simulink model "CdsnProbltyMgr"  (R2022b)
settings: Solver=FixedStepDiscrete  StartTime=0.0  StopTime=10.0  FixedStep=0.05  RelTol=1e-3
(part 2 of 2)

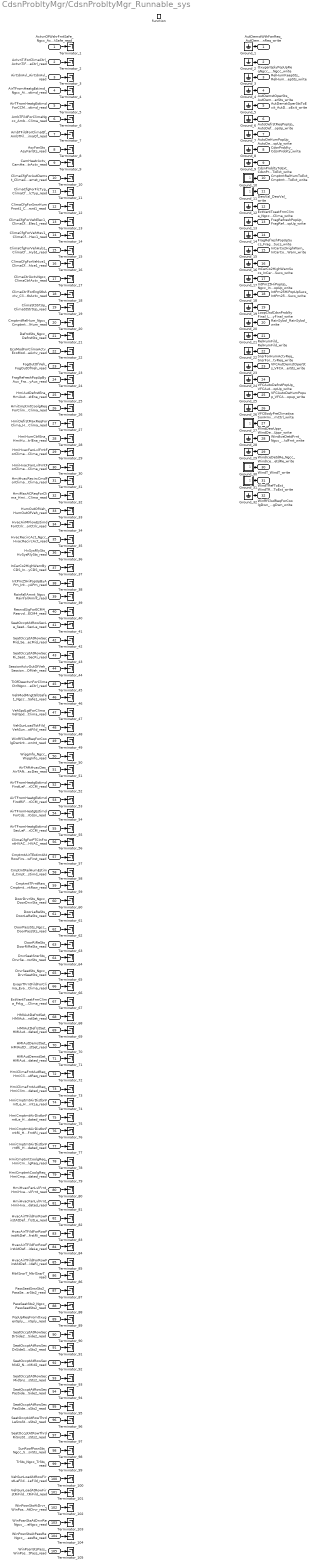
== system CdsnProbltyMgr/CdsnProbltyMgr_Runnable_sys ==
  ActvnOfWshrFrntSafe_Ngcc_ActvnOfWshrFrntSafe_read : Inport
  ActvnTiForClimaCtrl_ActvnTiForClimaCtrl_read : Inport  Port=2
  AirCdnAvl_AirCdnAvl_read : Inport  Port=3
  AirTFromHeatgEstimd_Ngcc_AirTFromHeatgEstimd_read : Inport  Port=4
  AirTFromHeatgEstimdForCCM_AirTFromHeatgEstimd_read : Inport  Port=5
  AmbTFildForClimaNgcc_AmbTFildForClima_read : Inport  Port=6
  AmbTFildForClimaQf_AmbTFildForClimaQf_read : Inport  Port=7
  AsyFanSts_AsyFanSts_read : Inport  Port=8
  CamHeatrActv_CamHeatrActv_read : Inport  Port=9
  ClimaCfgForAutDemst_ClimaCfgForAutDemst_read : Inport  Port=10
  ClimaCfgForFlcTyp_ClimaCfgForFlcTyp_read : Inport  Port=11
  ClimaCfgForSnsrHumPrsnt1_ClimaCfgForSnsrHumPrsnt1_read : Inport  Port=12
  ClimaCfgForVehElec1_ClimaCfgForVehElec1_read : Inport  Port=13
  ClimaCfgForVehHev1_ClimaCfgForVehHev1_read : Inport  Port=14
  ClimaCfgForVehHyb1_ClimaCfgForVehHyb1_read : Inport  Port=15
  ClimaCfgForVehIce1_ClimaCfgForVehIce1_read : Inport  Port=16
  ClimaCtrlActvNgcc_ClimaCtrlActv_read : Inport  Port=17
  ClimaCtrlForEngStbActv_ClimaCtrlForEngStbActv_read : Inport  Port=18
  ClimaStStrtUp_ClimaStStrtUp_read : Inport  Port=19
  CmptmtRelHum_Ngcc_CmptmtRelHum_read : Inport  Port=20
  DefrstSts_Ngcc_DefrstSts_read : Inport  Port=21
  EcoModForClimaActv_EcoModForClimaActv_read : Inport  Port=22
  FogOutOfVeh_FogOutOfVeh_read : Inport  Port=23
  FragRefreshPopUpByAux_FragRefreshPopUpByAux_read : Inport  Port=24
  HmiAutDefrstEna_HmiAutDefrstEna_read : Inport  Port=25
  HmiCmptmtCoolgReqForClima_HmiCmptmtCoolgReqForClima_read : Inport  Port=26
  HmiDefrstMaxReqForClima_HmiDefrstMaxReqForClima_read : Inport  Port=27
  HmiHumCtrlEna_HmiHumCtrlEna_read : Inport  Port=28
  HmiHvacFanLvlFrntForClima_HmiHvacFanLvlFrntForClima_read : Inport  Port=29
  HmiHvacFanLvlFrntForClima_HmiHvacFanLvlFrntRiForClima_read : Inport  Port=30
  HmiHvacRecircCmdForClima_HmiHvacRecircCmdForClima_read : Inport  Port=31
  HmiMaxACReqForClima_HmiMaxACReqForClima_read : Inport  Port=32
  HumOutOfVeh_HumOutOfVeh_read : Inport  Port=33
  HvacAirMFlowEstimdForCtrlr_HvacAirMFlowEstimdForCtrlr_read : Inport  Port=34
  HvacRecircAct_Ngcc_HvacRecircAct_read : Inport  Port=35
  HvSysRlySts_HvSysRlySts_read : Inport  Port=36
  InCarCo2HighWarnByCDS_InCarCo2HighWarnByCDS_read : Inport  Port=37
  IntPm25HiPopUpByAPm_IntPm25HiPopUpByAPm_read : Inport  Port=38
  RainfallAmnt_Ngcc_RainfallAmnt_read : Inport  Port=39
  ResrvdSigForECM4_ResrvdSigForECM4_read : Inport  Port=40
  SeatOccptAtRowSecLe_SeatOccptAtRowSecLe_read : Inport  Port=41
  SeatOccptAtRowSecMid_SeatOccptAtRowSecMid_read : Inport  Port=42
  SeatOccptAtRowSecRi_SeatOccptAtRowSecRi_read : Inport  Port=43
  SessionActvOutOfVeh_SessionActvOutOfVeh_read : Inport  Port=44
  TiOfDeactvnForClimaCtrlNgcc_TiOfDeactvnForClimaCtrl_read : Inport  Port=45
  VehModMngtGlbSafe1_Ngcc_VehModMngtGlbSafe1_read : Inport  Port=46
  VehSpdLgtForClima_VehSpdLgtForClima_read : Inport  Port=47
  VehSunLoadTotFild_VehSunLoadTotFild_read : Inport  Port=48
  WinRfClsdReqForCoolgDwnInt_WinRfClsdReqForCoolgDwnInt_read : Inport  Port=49
  WipgInfo_Ngcc_WipgInfo_read : Inport  Port=50
  AirTAftHvacDes_AirTAftHvacDes_read : Inport  Port=51
  AirTFromHeatgEstimdFirstLeForCCM_AirTFromHeatgEstimdFirstLeForCCM_read : Inport  Port=52
  AirTFromHeatgEstimdFirstRiForCCM_AirTFromHeatgEstimdFirstRiForCCM_read : Inport  Port=53
  AirTFromHeatgEstimdForCdsn_AirTFromHeatgEstimdForCdsn_read : Inport  Port=54
  AirTFromHeatgEstimdSecLeForCCM_AirTFromHeatgEstimdSecLeForCCM_read : Inport  Port=55
  ClimaCfgForPTCInFrontHVAC_ClimaCfgForPTCInFrontHVAC_read : Inport  Port=56
  CmptmtAirTEstimdAtRowFirst_CmptmtAirTEstimdAtRowFirst_read : Inport  Port=57
  CmptmtRelHumEstimd_CmptmtRelHumEstimd_read : Inport  Port=58
  CmptmtTFrntRaw_CmptmtTFrntRaw_read : Inport  Port=59
  DoorDrvrSts_Ngcc_DoorDrvrSts_read : Inport  Port=60
  DoorLeReSts_DoorLeReSts_read : Inport  Port=61
  DoorPassSts_Ngcc_DoorPassSts_read : Inport  Port=62
  DoorRiReSts_DoorRiReSts_read : Inport  Port=63
  DrvrSeatSnsrSts_DrvrSeatSnsrSts_read : Inport  Port=64
  DrvrSeatSts_Ngcc_DrvrSeatSts_read : Inport  Port=65
  EvaprTFrntFildForClima_EvaprTFrntFildForClima_read : Inport  Port=66
  ExtVentToastFrmClima_Prkg_ExtVentToastFrmClima_read : Inport  Port=67
  HMIAutDefrstSet_HMIAutDefrstSet_read : Inport  Port=68
  HMIAutDefrstSet_HMIAutDefrstSet_IsUpdated_read : Inport  Port=69
  HMIAutDemstSet_HMIAutDemstSet_read : Inport  Port=70
  HMIAutDemstSet_HMIAutDemstSet_IsUpdated_read : Inport  Port=71
  HmiClimaFrntAutReq_HmiClimaFrntAutReq_read : Inport  Port=72
  HmiClimaFrntAutReq_HmiClimaFrntAutReq_IsUpdated_read : Inport  Port=73
  HmiCmptmtAirDistbnFrntLe_HmiCmptmtAirDistbnFrntLe_read : Inport  Port=74
  HmiCmptmtAirDistbnFrntLe_HmiCmptmtAirDistbnFrntLe_IsUpdated_read : Inport  Port=75
  HmiCmptmtAirDistbnFrntRi_HmiCmptmtAirDistbnFrntRi_read : Inport  Port=76
  HmiCmptmtAirDistbnFrntRi_HmiCmptmtAirDistbnFrntRi_IsUpdated_read : Inport  Port=77
  HmiCmptmtCoolgReq_HmiCmptmtCoolgReq_read : Inport  Port=78
  HmiCmptmtCoolgReq_HmiCmptmtCoolgReq_IsUpdated_read : Inport  Port=79
  HmiHvacFanLvlFrnt_HmiHvacFanLvlFrnt_read : Inport  Port=80
  HmiHvacFanLvlFrnt_HmiHvacFanLvlFrnt_IsUpdated_read : Inport  Port=81
  HvacAirTFildForRowFirstAtDefrstLe_HvacAirTFildForRowFirstAtDefrstLe_read : Inport  Port=82
  HvacAirTFildForRowFirstAtDefrstRi_HvacAirTFildForRowFirstAtDefrstRi_read : Inport  Port=83
  HvacAirTFildForRowFirstAtDefrstSideLe_HvacAirTFildForRowFirstAtDefrstSideLe_read : Inport  Port=84
  HvacAirTFildForRowFirstAtDefrstSideRi_HvacAirTFildForRowFirstAtDefrstSideRi_read : Inport  Port=85
  MtrlSnsrT_MtrlSnsrT_read : Inport  Port=86
  PassSeatSnsrSts2_PassSeatSnsrSts2_read : Inport  Port=87
  PassSeatSts2_Ngcc_PassSeatSts2_read : Inport  Port=88
  PopUpReqFromOxygenSply_PopUpReqFromOxygenSply_read : Inport  Port=89
  SeatOccptAtRowSecDrSide2_Ngcc_SeatOccptAtRowSecDrSide2_read : Inport  Port=90
  SeatOccptAtRowSecDrSideSnsSts2_SeatOccptAtRowSecDrSideSnsSts2_read : Inport  Port=91
  SeatOccptAtRowSecMid2_Ngcc_SeatOccptAtRowSecMid2_read : Inport  Port=92
  SeatOccptAtRowSecMidSnsSts2_SeatOccptAtRowSecMidSnsSts2_read : Inport  Port=93
  SeatOccptAtRowSecPasSide2_Ngcc_SeatOccptAtRowSecPasSide2_read : Inport  Port=94
  SeatOccptAtRowSecPasSideSnsSts2_SeatOccptAtRowSecPasSideSnsSts2_read : Inport  Port=95
  SeatOccptAtRowThrdLeSnsSts2_SeatOccptAtRowThrdLeSnsSts2_read : Inport  Port=96
  SeatOccptAtRowThrdRiSnsSts2_SeatOccptAtRowThrdRiSnsSts2_read : Inport  Port=97
  SunRoofPosnSts_Ngcc_SunRoofPosnSts_read : Inport  Port=98
  TrSts_Ngcc_TrSts_read : Inport  Port=99
  VehSunLoadAtRowFirstLeFild_VehSunLoadAtRowFirstLeFild_read : Inport  Port=100
  VehSunLoadAtRowFirstRiFild_VehSunLoadAtRowFirstRiFild_read : Inport  Port=101
  WinPosnStsAtDrvr_WinPosnStsAtDrvr_read : Inport  Port=102
  WinPosnStsAtDrvrReNgcc_WinPosnStsAtDrvrReNgcc_read : Inport  Port=103
  WinPosnStsAtPassReNgcc_WinPosnStsAtPassRe_read : Inport  Port=104
  WinPosnStsPass_WinPosnStsAtPass_read : Inport  Port=105
  function : TriggerPort  TriggerType=function-call  VariantControl=(inherit)  SampleTimeType=periodic  SampleTime=-1
  Ground_{1..9,12..26,28..29,32} (27 alike) : Ground
  Ground_10 : Subsystem
  Ground_11 : Subsystem
  Ground_27 : Subsystem
  Ground_30 : Subsystem
  Ground_31 : Subsystem
  Terminator_{1..105} (105 alike) : Terminator
  AutDemstWthFanReq_AutDemstWthFanReq_write : Outport
      settings: EnsureOutportIsVirtual=on
  OxygenSplyPopUpReqNgcc_OxygenSplyPopUpReqNgcc_write : Outport  Port=2
      settings: EnsureOutportIsVirtual=on
  RelHumKeepSts_RelHumKeepSts_write : Outport  Port=3
      settings: EnsureOutportIsVirtual=on
  AutDemstOperSts_AutDemstOperSts_write : Outport  Port=4
      settings: EnsureOutportIsVirtual=on
  AutDemstOperStsToExit_AutDemstOperStsToExit_write : Outport  Port=5
      settings: EnsureOutportIsVirtual=on
  AutoDefrstReqPopUp_AutoDefrstReqPopUp_write : Outport  Port=6
      settings: EnsureOutportIsVirtual=on
  AutoDeHumPopUp_AutoDeHumPopUp_write : Outport  Port=7
      settings: EnsureOutportIsVirtual=on
  CdsnProblty_CdsnProblty_write : Outport  Port=8
      settings: EnsureOutportIsVirtual=on
  CdsnProbltyToExt_CdsnProbltyToExt_write : Outport  Port=9
      settings: EnsureOutportIsVirtual=on
  CmptmtRelHumToExt_CmptmtRelHumToExt_write : Outport  Port=10
      settings: EnsureOutportIsVirtual=on
  DewVal_DewVal_write : Outport  Port=11
      settings: EnsureOutportIsVirtual=on
  ExtVentToastFrmClima_Ngcc_ExtVentToastFrmClima_write : Outport  Port=12
      settings: EnsureOutportIsVirtual=on
  FragRefreshPopUp_FragRefreshPopUp_write : Outport  Port=13
      settings: EnsureOutportIsVirtual=on
  FragRefreshPopUpSucs_FragRefreshPopUpSucs_write : Outport  Port=14
      settings: EnsureOutportIsVirtual=on
  InCarCo2HighWarn_InCarCo2HighWarn_write : Outport  Port=15
      settings: EnsureOutportIsVirtual=on
  InCarCo2HighWarnSucs_InCarCo2HighWarnSucs_write : Outport  Port=16
      settings: EnsureOutportIsVirtual=on
  IntPm25HiPopUp_Ngcc_IntPm25HiPopUp_write : Outport  Port=17
      settings: EnsureOutportIsVirtual=on
  IntPm25HiPopUpSucs_IntPm25HiPopUpSucs_write : Outport  Port=18
      settings: EnsureOutportIsVirtual=on
  LoopClsdCdsnProbltyFinal_LoopClsdCdsnProbltyFinal_write : Outport  Port=19
      settings: EnsureOutportIsVirtual=on
  RainSybol_RainSybol_write : Outport  Port=20
      settings: EnsureOutportIsVirtual=on
  RelHumFild_RelHumFild_write : Outport  Port=21
      settings: EnsureOutportIsVirtual=on
  SnsrForHumActvReq_SnsrForHumActvReq_write : Outport  Port=22
      settings: EnsureOutportIsVirtual=on
  VFCAutDemstOperSts_VFCAutDemstOperSts_write : Outport  Port=23
      settings: EnsureOutportIsVirtual=on
  VFCAutoDefrstPopUp_VFCAutoDefrstPopUp_write : Outport  Port=24
      settings: EnsureOutportIsVirtual=on
  VFCAutoDeHumPopup_VFCAutoDeHumPopup_write : Outport  Port=25
      settings: EnsureOutportIsVirtual=on
  VFCBodyPreClimatisationHmiHumCtrl_VFCBodyPreClimatisationHmiHumCtrl_write : Outport  Port=26
      settings: EnsureOutportIsVirtual=on
  WindDewUppr_WindDewTUppr_write : Outport  Port=27
      settings: EnsureOutportIsVirtual=on
  WindIceDetdFrnt_Ngcc_WindIceDetdFrnt_write : Outport  Port=28
      settings: EnsureOutportIsVirtual=on
  WindIceDetdRe_Ngcc_WindIceDetdRe_write : Outport  Port=29
      settings: EnsureOutportIsVirtual=on
  WindT_WindT_write : Outport  Port=30
      settings: EnsureOutportIsVirtual=on
  WindTRefToExt_WindTRefToExt_write : Outport  Port=31
      settings: EnsureOutportIsVirtual=on
  WinRfClsdReqForCoolgDwn_WinRfClsdReqForCoolgDwn_write : Outport  Port=32
      settings: EnsureOutportIsVirtual=on
  wires:
  ActvnOfWshrFrntSafe_Ngcc_ActvnOfWshrFrntSafe_read/1 -> Terminator_1/1
  ActvnTiForClimaCtrl_ActvnTiForClimaCtrl_read/1 -> Terminator_2/1
  AirCdnAvl_AirCdnAvl_read/1 -> Terminator_3/1
  AirTFromHeatgEstimd_Ngcc_AirTFromHeatgEstimd_read/1 -> Terminator_4/1
  AirTFromHeatgEstimdForCCM_AirTFromHeatgEstimd_read/1 -> Terminator_5/1
  AmbTFildForClimaNgcc_AmbTFildForClima_read/1 -> Terminator_6/1
  AmbTFildForClimaQf_AmbTFildForClimaQf_read/1 -> Terminator_7/1
  AsyFanSts_AsyFanSts_read/1 -> Terminator_8/1
  CamHeatrActv_CamHeatrActv_read/1 -> Terminator_9/1
  ClimaCfgForAutDemst_ClimaCfgForAutDemst_read/1 -> Terminator_10/1
  ClimaCfgForFlcTyp_ClimaCfgForFlcTyp_read/1 -> Terminator_11/1
  ClimaCfgForSnsrHumPrsnt1_ClimaCfgForSnsrHumPrsnt1_read/1 -> Terminator_12/1
  ClimaCfgForVehElec1_ClimaCfgForVehElec1_read/1 -> Terminator_13/1
  ClimaCfgForVehHev1_ClimaCfgForVehHev1_read/1 -> Terminator_14/1
  ClimaCfgForVehHyb1_ClimaCfgForVehHyb1_read/1 -> Terminator_15/1
  ClimaCfgForVehIce1_ClimaCfgForVehIce1_read/1 -> Terminator_16/1
  ClimaCtrlActvNgcc_ClimaCtrlActv_read/1 -> Terminator_17/1
  ClimaCtrlForEngStbActv_ClimaCtrlForEngStbActv_read/1 -> Terminator_18/1
  ClimaStStrtUp_ClimaStStrtUp_read/1 -> Terminator_19/1
  CmptmtRelHum_Ngcc_CmptmtRelHum_read/1 -> Terminator_20/1
  DefrstSts_Ngcc_DefrstSts_read/1 -> Terminator_21/1
  EcoModForClimaActv_EcoModForClimaActv_read/1 -> Terminator_22/1
  FogOutOfVeh_FogOutOfVeh_read/1 -> Terminator_23/1
  FragRefreshPopUpByAux_FragRefreshPopUpByAux_read/1 -> Terminator_24/1
  HmiAutDefrstEna_HmiAutDefrstEna_read/1 -> Terminator_25/1
  HmiCmptmtCoolgReqForClima_HmiCmptmtCoolgReqForClima_read/1 -> Terminator_26/1
  HmiDefrstMaxReqForClima_HmiDefrstMaxReqForClima_read/1 -> Terminator_27/1
  HmiHumCtrlEna_HmiHumCtrlEna_read/1 -> Terminator_28/1
  HmiHvacFanLvlFrntForClima_HmiHvacFanLvlFrntForClima_read/1 -> Terminator_29/1
  HmiHvacFanLvlFrntForClima_HmiHvacFanLvlFrntRiForClima_read/1 -> Terminator_30/1
  HmiHvacRecircCmdForClima_HmiHvacRecircCmdForClima_read/1 -> Terminator_31/1
  HmiMaxACReqForClima_HmiMaxACReqForClima_read/1 -> Terminator_32/1
  HumOutOfVeh_HumOutOfVeh_read/1 -> Terminator_33/1
  HvacAirMFlowEstimdForCtrlr_HvacAirMFlowEstimdForCtrlr_read/1 -> Terminator_34/1
  HvacRecircAct_Ngcc_HvacRecircAct_read/1 -> Terminator_35/1
  HvSysRlySts_HvSysRlySts_read/1 -> Terminator_36/1
  InCarCo2HighWarnByCDS_InCarCo2HighWarnByCDS_read/1 -> Terminator_37/1
  IntPm25HiPopUpByAPm_IntPm25HiPopUpByAPm_read/1 -> Terminator_38/1
  RainfallAmnt_Ngcc_RainfallAmnt_read/1 -> Terminator_39/1
  ResrvdSigForECM4_ResrvdSigForECM4_read/1 -> Terminator_40/1
  SeatOccptAtRowSecLe_SeatOccptAtRowSecLe_read/1 -> Terminator_41/1
  SeatOccptAtRowSecMid_SeatOccptAtRowSecMid_read/1 -> Terminator_42/1
  SeatOccptAtRowSecRi_SeatOccptAtRowSecRi_read/1 -> Terminator_43/1
  SessionActvOutOfVeh_SessionActvOutOfVeh_read/1 -> Terminator_44/1
  TiOfDeactvnForClimaCtrlNgcc_TiOfDeactvnForClimaCtrl_read/1 -> Terminator_45/1
  VehModMngtGlbSafe1_Ngcc_VehModMngtGlbSafe1_read/1 -> Terminator_46/1
  VehSpdLgtForClima_VehSpdLgtForClima_read/1 -> Terminator_47/1
  VehSunLoadTotFild_VehSunLoadTotFild_read/1 -> Terminator_48/1
  WinRfClsdReqForCoolgDwnInt_WinRfClsdReqForCoolgDwnInt_read/1 -> Terminator_49/1
  WipgInfo_Ngcc_WipgInfo_read/1 -> Terminator_50/1
  AirTAftHvacDes_AirTAftHvacDes_read/1 -> Terminator_51/1
  AirTFromHeatgEstimdFirstLeForCCM_AirTFromHeatgEstimdFirstLeForCCM_read/1 -> Terminator_52/1
  AirTFromHeatgEstimdFirstRiForCCM_AirTFromHeatgEstimdFirstRiForCCM_read/1 -> Terminator_53/1
  AirTFromHeatgEstimdForCdsn_AirTFromHeatgEstimdForCdsn_read/1 -> Terminator_54/1
  AirTFromHeatgEstimdSecLeForCCM_AirTFromHeatgEstimdSecLeForCCM_read/1 -> Terminator_55/1
  ClimaCfgForPTCInFrontHVAC_ClimaCfgForPTCInFrontHVAC_read/1 -> Terminator_56/1
  CmptmtAirTEstimdAtRowFirst_CmptmtAirTEstimdAtRowFirst_read/1 -> Terminator_57/1
  CmptmtRelHumEstimd_CmptmtRelHumEstimd_read/1 -> Terminator_58/1
  CmptmtTFrntRaw_CmptmtTFrntRaw_read/1 -> Terminator_59/1
  DoorDrvrSts_Ngcc_DoorDrvrSts_read/1 -> Terminator_60/1
  DoorLeReSts_DoorLeReSts_read/1 -> Terminator_61/1
  DoorPassSts_Ngcc_DoorPassSts_read/1 -> Terminator_62/1
  DoorRiReSts_DoorRiReSts_read/1 -> Terminator_63/1
  DrvrSeatSnsrSts_DrvrSeatSnsrSts_read/1 -> Terminator_64/1
  DrvrSeatSts_Ngcc_DrvrSeatSts_read/1 -> Terminator_65/1
  EvaprTFrntFildForClima_EvaprTFrntFildForClima_read/1 -> Terminator_66/1
  ExtVentToastFrmClima_Prkg_ExtVentToastFrmClima_read/1 -> Terminator_67/1
  HMIAutDefrstSet_HMIAutDefrstSet_read/1 -> Terminator_68/1
  HMIAutDefrstSet_HMIAutDefrstSet_IsUpdated_read/1 -> Terminator_69/1
  HMIAutDemstSet_HMIAutDemstSet_read/1 -> Terminator_70/1
  HMIAutDemstSet_HMIAutDemstSet_IsUpdated_read/1 -> Terminator_71/1
  HmiClimaFrntAutReq_HmiClimaFrntAutReq_read/1 -> Terminator_72/1
  HmiClimaFrntAutReq_HmiClimaFrntAutReq_IsUpdated_read/1 -> Terminator_73/1
  HmiCmptmtAirDistbnFrntLe_HmiCmptmtAirDistbnFrntLe_read/1 -> Terminator_74/1
  HmiCmptmtAirDistbnFrntLe_HmiCmptmtAirDistbnFrntLe_IsUpdated_read/1 -> Terminator_75/1
  HmiCmptmtAirDistbnFrntRi_HmiCmptmtAirDistbnFrntRi_read/1 -> Terminator_76/1
  HmiCmptmtAirDistbnFrntRi_HmiCmptmtAirDistbnFrntRi_IsUpdated_read/1 -> Terminator_77/1
  HmiCmptmtCoolgReq_HmiCmptmtCoolgReq_read/1 -> Terminator_78/1
  HmiCmptmtCoolgReq_HmiCmptmtCoolgReq_IsUpdated_read/1 -> Terminator_79/1
  HmiHvacFanLvlFrnt_HmiHvacFanLvlFrnt_read/1 -> Terminator_80/1
  HmiHvacFanLvlFrnt_HmiHvacFanLvlFrnt_IsUpdated_read/1 -> Terminator_81/1
  HvacAirTFildForRowFirstAtDefrstLe_HvacAirTFildForRowFirstAtDefrstLe_read/1 -> Terminator_82/1
  HvacAirTFildForRowFirstAtDefrstRi_HvacAirTFildForRowFirstAtDefrstRi_read/1 -> Terminator_83/1
  HvacAirTFildForRowFirstAtDefrstSideLe_HvacAirTFildForRowFirstAtDefrstSideLe_read/1 -> Terminator_84/1
  HvacAirTFildForRowFirstAtDefrstSideRi_HvacAirTFildForRowFirstAtDefrstSideRi_read/1 -> Terminator_85/1
  MtrlSnsrT_MtrlSnsrT_read/1 -> Terminator_86/1
  PassSeatSnsrSts2_PassSeatSnsrSts2_read/1 -> Terminator_87/1
  PassSeatSts2_Ngcc_PassSeatSts2_read/1 -> Terminator_88/1
  PopUpReqFromOxygenSply_PopUpReqFromOxygenSply_read/1 -> Terminator_89/1
  SeatOccptAtRowSecDrSide2_Ngcc_SeatOccptAtRowSecDrSide2_read/1 -> Terminator_90/1
  SeatOccptAtRowSecDrSideSnsSts2_SeatOccptAtRowSecDrSideSnsSts2_read/1 -> Terminator_91/1
  SeatOccptAtRowSecMid2_Ngcc_SeatOccptAtRowSecMid2_read/1 -> Terminator_92/1
  SeatOccptAtRowSecMidSnsSts2_SeatOccptAtRowSecMidSnsSts2_read/1 -> Terminator_93/1
  SeatOccptAtRowSecPasSide2_Ngcc_SeatOccptAtRowSecPasSide2_read/1 -> Terminator_94/1
  SeatOccptAtRowSecPasSideSnsSts2_SeatOccptAtRowSecPasSideSnsSts2_read/1 -> Terminator_95/1
  SeatOccptAtRowThrdLeSnsSts2_SeatOccptAtRowThrdLeSnsSts2_read/1 -> Terminator_96/1
  SeatOccptAtRowThrdRiSnsSts2_SeatOccptAtRowThrdRiSnsSts2_read/1 -> Terminator_97/1
  SunRoofPosnSts_Ngcc_SunRoofPosnSts_read/1 -> Terminator_98/1
  TrSts_Ngcc_TrSts_read/1 -> Terminator_99/1
  VehSunLoadAtRowFirstLeFild_VehSunLoadAtRowFirstLeFild_read/1 -> Terminator_100/1
  VehSunLoadAtRowFirstRiFild_VehSunLoadAtRowFirstRiFild_read/1 -> Terminator_101/1
  WinPosnStsAtDrvr_WinPosnStsAtDrvr_read/1 -> Terminator_102/1
  WinPosnStsAtDrvrReNgcc_WinPosnStsAtDrvrReNgcc_read/1 -> Terminator_103/1
  WinPosnStsAtPassReNgcc_WinPosnStsAtPassRe_read/1 -> Terminator_104/1
  WinPosnStsPass_WinPosnStsAtPass_read/1 -> Terminator_105/1
  Ground_1/1 -> AutDemstWthFanReq_AutDemstWthFanReq_write/1
  Ground_2/1 -> OxygenSplyPopUpReqNgcc_OxygenSplyPopUpReqNgcc_write/1
  Ground_3/1 -> RelHumKeepSts_RelHumKeepSts_write/1
  Ground_4/1 -> AutDemstOperSts_AutDemstOperSts_write/1
  Ground_5/1 -> AutDemstOperStsToExit_AutDemstOperStsToExit_write/1
  Ground_6/1 -> AutoDefrstReqPopUp_AutoDefrstReqPopUp_write/1
  Ground_7/1 -> AutoDeHumPopUp_AutoDeHumPopUp_write/1
  Ground_8/1 -> CdsnProblty_CdsnProblty_write/1
  Ground_9/1 -> CdsnProbltyToExt_CdsnProbltyToExt_write/1
  Ground_10/1(Out1) -> CmptmtRelHumToExt_CmptmtRelHumToExt_write/1
  Ground_11/1(Out1) -> DewVal_DewVal_write/1
  Ground_12/1 -> ExtVentToastFrmClima_Ngcc_ExtVentToastFrmClima_write/1
  Ground_13/1 -> FragRefreshPopUp_FragRefreshPopUp_write/1
  Ground_14/1 -> FragRefreshPopUpSucs_FragRefreshPopUpSucs_write/1
  Ground_15/1 -> InCarCo2HighWarn_InCarCo2HighWarn_write/1
  Ground_16/1 -> InCarCo2HighWarnSucs_InCarCo2HighWarnSucs_write/1
  Ground_17/1 -> IntPm25HiPopUp_Ngcc_IntPm25HiPopUp_write/1
  Ground_18/1 -> IntPm25HiPopUpSucs_IntPm25HiPopUpSucs_write/1
  Ground_19/1 -> LoopClsdCdsnProbltyFinal_LoopClsdCdsnProbltyFinal_write/1
  Ground_20/1 -> RainSybol_RainSybol_write/1
  Ground_21/1 -> RelHumFild_RelHumFild_write/1
  Ground_22/1 -> SnsrForHumActvReq_SnsrForHumActvReq_write/1
  Ground_23/1 -> VFCAutDemstOperSts_VFCAutDemstOperSts_write/1
  Ground_24/1 -> VFCAutoDefrstPopUp_VFCAutoDefrstPopUp_write/1
  Ground_25/1 -> VFCAutoDeHumPopup_VFCAutoDeHumPopup_write/1
  Ground_26/1 -> VFCBodyPreClimatisationHmiHumCtrl_VFCBodyPreClimatisationHmiHumCtrl_write/1
  Ground_27/1(Out1) -> WindDewUppr_WindDewTUppr_write/1
  Ground_28/1 -> WindIceDetdFrnt_Ngcc_WindIceDetdFrnt_write/1
  Ground_29/1 -> WindIceDetdRe_Ngcc_WindIceDetdRe_write/1
  Ground_30/1(Out1) -> WindT_WindT_write/1
  Ground_31/1(Out1) -> WindTRefToExt_WindTRefToExt_write/1
  Ground_32/1 -> WinRfClsdReqForCoolgDwn_WinRfClsdReqForCoolgDwn_write/1

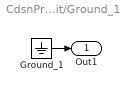
== system CdsnProbltyMgr/CdsnProbltyMgr_Init/Ground_1 ==
  Ground_1 : Ground
  Out1 : Outport  OutDataTypeStr=Bus: AirDewMeasValReq  PortDimensions=1
  wires:
  Ground_1/1 -> Out1/1

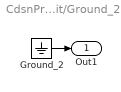
== system CdsnProbltyMgr/CdsnProbltyMgr_Init/Ground_2 ==
  Ground_2 : Ground
  Out1 : Outport  OutDataTypeStr=Bus: WindDewTUppr  PortDimensions=1
  wires:
  Ground_2/1 -> Out1/1

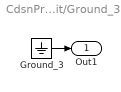
== system CdsnProbltyMgr/CdsnProbltyMgr_Init/Ground_3 ==
  Ground_3 : Ground
  Out1 : Outport  OutDataTypeStr=Bus: WindT  PortDimensions=1
  wires:
  Ground_3/1 -> Out1/1

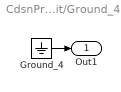
== system CdsnProbltyMgr/CdsnProbltyMgr_Init/Ground_4 ==
  Ground_4 : Ground
  Out1 : Outport  OutDataTypeStr=Bus: WindT  PortDimensions=1
  wires:
  Ground_4/1 -> Out1/1

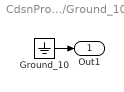
== system CdsnProbltyMgr/CdsnProbltyMgr_Runnable_sys/Ground_10 ==
  Ground_10 : Ground
  Out1 : Outport  OutDataTypeStr=Bus: CmptmtRelHumToExt  PortDimensions=1
  wires:
  Ground_10/1 -> Out1/1

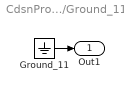
== system CdsnProbltyMgr/CdsnProbltyMgr_Runnable_sys/Ground_11 ==
  Ground_11 : Ground
  Out1 : Outport  OutDataTypeStr=Bus: AirDewMeasValReq  PortDimensions=1
  wires:
  Ground_11/1 -> Out1/1

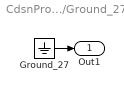
== system CdsnProbltyMgr/CdsnProbltyMgr_Runnable_sys/Ground_27 ==
  Ground_27 : Ground
  Out1 : Outport  OutDataTypeStr=Bus: WindDewTUppr  PortDimensions=1
  wires:
  Ground_27/1 -> Out1/1

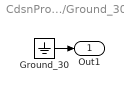
== system CdsnProbltyMgr/CdsnProbltyMgr_Runnable_sys/Ground_30 ==
  Ground_30 : Ground
  Out1 : Outport  OutDataTypeStr=Bus: WindT  PortDimensions=1
  wires:
  Ground_30/1 -> Out1/1

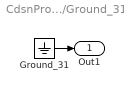
== system CdsnProbltyMgr/CdsnProbltyMgr_Runnable_sys/Ground_31 ==
  Ground_31 : Ground
  Out1 : Outport  OutDataTypeStr=Bus: WindT  PortDimensions=1
  wires:
  Ground_31/1 -> Out1/1
